simulink model "isi_correlator"  (Simulink 7.0)
settings: Solver=ode45  StartTime=0.0  StopTime=2500  RelTol=1e-3
undefined symbols (not defined in the file): bit_rev, pfb_coeff_gen_calc
libraries: xbsIndex_r4 x4490, casper_library x399, xbsReplacements_r4 x27, isi_correlator_lib x8, xps_library x7, dspsigops x2, simulink x1
(part 1 of 49)
settings Model: Creator=wjm  LastModifiedBy=wjm

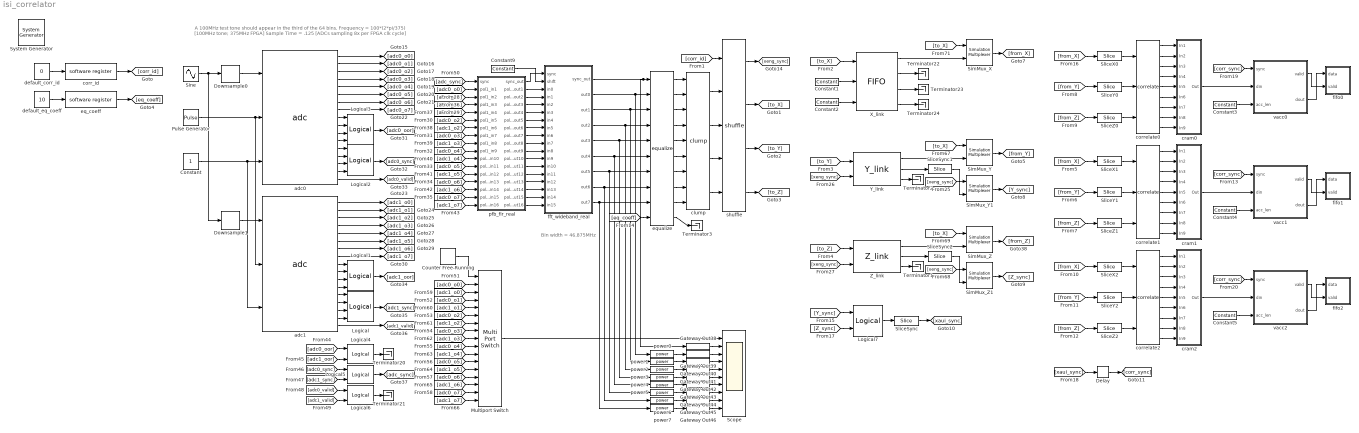
== system isi_correlator ==
  note: A 100MHz test tone should appear in the third of the 64 bins.
      | Frequency = 100*(2*pi/375) [100MHz tone; 375MHz FPGA]
      | Sample Time = .125 [ADCs sampling 8x per FPGA clk cycle]
  note: Bin width = 46.875MHz
  " System Generator" : xbsIndex_r4/ System Generator  Tag=genX  SystemSampleTime=-1  xilinxfamily=virtex5  part=xc5vsx95t  speed=-1  package=ff1136  synthesis_tool=XST  clock_wrapper=Clock Enables  directory=./isi_correlator/sysgen  testbench=off  simulink_period=1  sysclk_period=2.6667  dcm_input_clock_period=100  incr_netlist=off  trim_vbits=Everywhere in SubSystem  dbl_ovrd=According to Block Masks  core_generation=According to Block Masks  run_coregen=off  deprecated_control=off  eval_field=0  block_type=sysgen
      settings: block_version=10.1.1
  Constant : Constant  OutDataType=sfix(16)  OutScaling=2^0  SampleTime=1
  Constant1 : xbsIndex_r4/Constant  arith_type=Boolean  const=1  n_bits=1  bin_pt=0  explicit_period=on  period=1  equ=P=C  opselect=C  inp2=PCIN>>17  opr=+  inp1=P  carry=CIN  dbl_ovrd=off  block_type=constant
      settings: block_version=10.1.1
  Constant2 : xbsIndex_r4/Constant  arith_type=Boolean  const=1  n_bits=1  bin_pt=0  explicit_period=on  period=1  equ=P=C  opselect=C  inp2=PCIN>>17  opr=+  inp1=P  carry=CIN  dbl_ovrd=off  block_type=constant
      settings: block_version=10.1.1
  Constant3 : xbsIndex_r4/Constant  arith_type=Unsigned  const=127  n_bits=8  bin_pt=0  explicit_period=on  period=1  equ=P=C  opselect=C  inp2=PCIN>>17  opr=+  inp1=P  carry=CIN  dbl_ovrd=off  block_type=constant
      settings: block_version=10.1.1
  Constant4 : xbsIndex_r4/Constant  arith_type=Unsigned  const=127  n_bits=8  bin_pt=0  explicit_period=on  period=1  equ=P=C  opselect=C  inp2=PCIN>>17  opr=+  inp1=P  carry=CIN  dbl_ovrd=off  block_type=constant
      settings: block_version=10.1.1
  Constant5 : xbsIndex_r4/Constant  arith_type=Unsigned  const=127  n_bits=8  bin_pt=0  explicit_period=on  period=1  equ=P=C  opselect=C  inp2=PCIN>>17  opr=+  inp1=P  carry=CIN  dbl_ovrd=off  block_type=constant
      settings: block_version=10.1.1
  Constant9 : xbsIndex_r4/Constant  arith_type=Unsigned  const=127  n_bits=7  bin_pt=0  explicit_period=on  period=1  equ=P=C  opselect=C  inp2=PCIN>>17  opr=+  inp1=P  carry=CIN  dbl_ovrd=off  block_type=constant
      settings: block_version=10.1.1
  Counter Free-Running : simulink/Sources/Counter Free-Running  SystemSampleTime=-1  NumBits=4  tsamp=1/16
  Delay : xbsIndex_r4/Delay  en=off  latency=1  dbl_ovrd=off  reg_retiming=off  block_type=delay
  Downsample0 : dspsigops/Downsample  N=2  phase=1  ic=0  smode=Allow multirate  fmode=Maintain input frame size
  Downsample1 : dspsigops/Downsample  N=2  phase=0  ic=0  smode=Allow multirate  fmode=Maintain input frame size
  Gateway Out{38..46} (9 alike) : xbsIndex_r4/Gateway Out  hdl_port=on  timing_constraint=None  locs_specified=off  LOCs={}  block_type=gatewayout
      settings: infoedit="Gateway out block.  Converts Xilinx fixed point inputs into ouputs of type Simulink integer, double, or fixed point.<P><P>Hardware notes:  In hardware these blocks become top level output ports or are discarded, depending on how they are configured."  block_version=10.1.3
  Logical{,1..3} (4 alike) : xbsIndex_r4/Logical  logical_function=OR  inputs=4  en=off  latency=0  precision=Full  arith_type=Unsigned  n_bits=1  bin_pt=0  align_bp=on  dbl_ovrd=off  block_type=logical
      settings: block_version=10.1.1
  Logical4 : xbsIndex_r4/Logical  logical_function=OR  inputs=2  en=off  latency=0  precision=Full  arith_type=Unsigned  n_bits=1  bin_pt=0  align_bp=on  dbl_ovrd=off  block_type=logical
      settings: block_version=10.1.1
  Logical5 : xbsIndex_r4/Logical  logical_function=OR  inputs=2  en=off  latency=0  precision=Full  arith_type=Unsigned  n_bits=1  bin_pt=0  align_bp=on  dbl_ovrd=off  block_type=logical
      settings: block_version=10.1.1
  Logical6 : xbsIndex_r4/Logical  logical_function=AND  inputs=2  en=off  latency=0  precision=Full  arith_type=Unsigned  n_bits=1  bin_pt=0  align_bp=on  dbl_ovrd=off  block_type=logical
      settings: block_version=10.1.1
  Logical7 : xbsIndex_r4/Logical  logical_function=OR  inputs=2  en=off  latency=0  precision=Full  arith_type=Unsigned  n_bits=16  bin_pt=0  align_bp=on  dbl_ovrd=off  block_type=logical
  Multiport Switch : MultiPortSwitch  Inputs=16  zeroidx=on  OutDataType=sfix(16)  OutScaling=2^0
  Pulse Generator : DiscretePulseGenerator  Period=2^12
  Scope : Scope  NumInputPorts=10
      settings: YMin=-1~-1~-1~0~-8.13152e-020~0~0~0~0~0  YMax=1~1~1~0.00275~0.0014~0.00175~0.00175~0.00225~0.00175~0.00225
  SimMux_X : xbsIndex_r4/Simulation Multiplexer  sim_sel=1  hw_sel=2  block_type=simmux
      settings: infoedit="Distinguish input subsystems as \"simulation only\" and \"generation only\".  The input specified For Simulation will be used during Simulink simulation.  The input specified For Generation will be used during code generation.  This block will typically be used on the output of a HW Co-Simulation, Black Box, or ModelSim subsystem.<P><P>Hardware Notes: This block costs nothing."  block_version=10.1.3
  SimMux_Y : xbsIndex_r4/Simulation Multiplexer  sim_sel=1  hw_sel=2  block_type=simmux
      settings: infoedit="Distinguish input subsystems as \"simulation only\" and \"generation only\".  The input specified For Simulation will be used during Simulink simulation.  The input specified For Generation will be used during code generation.  This block will typically be used on the output of a HW Co-Simulation, Black Box, or ModelSim subsystem.<P><P>Hardware Notes: This block costs nothing."  block_version=10.1.3
  SimMux_Y1 : xbsIndex_r4/Simulation Multiplexer  sim_sel=1  hw_sel=2  block_type=simmux
      settings: infoedit="Distinguish input subsystems as \"simulation only\" and \"generation only\".  The input specified For Simulation will be used during Simulink simulation.  The input specified For Generation will be used during code generation.  This block will typically be used on the output of a HW Co-Simulation, Black Box, or ModelSim subsystem.<P><P>Hardware Notes: This block costs nothing."  block_version=10.1.3
  SimMux_Z : xbsIndex_r4/Simulation Multiplexer  sim_sel=1  hw_sel=2  block_type=simmux
      settings: infoedit="Distinguish input subsystems as \"simulation only\" and \"generation only\".  The input specified For Simulation will be used during Simulink simulation.  The input specified For Generation will be used during code generation.  This block will typically be used on the output of a HW Co-Simulation, Black Box, or ModelSim subsystem.<P><P>Hardware Notes: This block costs nothing."  block_version=10.1.3
  SimMux_Z1 : xbsIndex_r4/Simulation Multiplexer  sim_sel=1  hw_sel=2  block_type=simmux
      settings: infoedit="Distinguish input subsystems as \"simulation only\" and \"generation only\".  The input specified For Simulation will be used during Simulink simulation.  The input specified For Generation will be used during code generation.  This block will typically be used on the output of a HW Co-Simulation, Black Box, or ModelSim subsystem.<P><P>Hardware Notes: This block costs nothing."  block_version=10.1.3
  Sine : Sin  Amplitude=.9  Frequency=100*(2*pi/375)  SampleTime=1/16
  SliceSync : xbsIndex_r4/Slice  nbits=8  boolean_output=on  mode=Lower Bit Location + Width  bit1=0  base1=MSB of Input  bit0=0  base0=LSB of Input  dbl_ovrd=off  block_type=slice
      settings: infoedit="Extracts a given range of bits from each input sample and presents it at the output.  The output type is ordinarily unsigned with binary point at zero, but can be Boolean when the slice is one bit wide.<P><P>Hardware notes: In hardware this block costs nothing."  block_version=10.1.1
  SliceSync1 : xbsIndex_r4/Slice  nbits=8  boolean_output=on  mode=Lower Bit Location + Width  bit1=0  base1=MSB of Input  bit0=0  base0=LSB of Input  dbl_ovrd=off  block_type=slice
      settings: infoedit="Extracts a given range of bits from each input sample and presents it at the output.  The output type is ordinarily unsigned with binary point at zero, but can be Boolean when the slice is one bit wide.<P><P>Hardware notes: In hardware this block costs nothing."  block_version=10.1.1
  SliceSync2 : xbsIndex_r4/Slice  nbits=8  boolean_output=on  mode=Lower Bit Location + Width  bit1=0  base1=MSB of Input  bit0=0  base0=LSB of Input  dbl_ovrd=off  block_type=slice
      settings: infoedit="Extracts a given range of bits from each input sample and presents it at the output.  The output type is ordinarily unsigned with binary point at zero, but can be Boolean when the slice is one bit wide.<P><P>Hardware notes: In hardware this block costs nothing."  block_version=10.1.1
  SliceX0 : xbsIndex_r4/Slice  nbits=8  boolean_output=off  mode=Upper Bit Location + Width  bit1=0  base1=MSB of Input  bit0=0  base0=LSB of Input  dbl_ovrd=off  block_type=slice
      settings: infoedit="Extracts a given range of bits from each input sample and presents it at the output.  The output type is ordinarily unsigned with binary point at zero, but can be Boolean when the slice is one bit wide.<P><P>Hardware notes: In hardware this block costs nothing."  block_version=10.1.1
  SliceX1 : xbsIndex_r4/Slice  nbits=8  boolean_output=off  mode=Upper Bit Location + Width  bit1=-8  base1=MSB of Input  bit0=8  base0=LSB of Input  dbl_ovrd=off  block_type=slice
      settings: infoedit="Extracts a given range of bits from each input sample and presents it at the output.  The output type is ordinarily unsigned with binary point at zero, but can be Boolean when the slice is one bit wide.<P><P>Hardware notes: In hardware this block costs nothing."  block_version=10.1.1
  SliceX2 : xbsIndex_r4/Slice  nbits=8  boolean_output=off  mode=Upper Bit Location + Width  bit1=-16  base1=MSB of Input  bit0=16  base0=LSB of Input  dbl_ovrd=off  block_type=slice
      settings: infoedit="Extracts a given range of bits from each input sample and presents it at the output.  The output type is ordinarily unsigned with binary point at zero, but can be Boolean when the slice is one bit wide.<P><P>Hardware notes: In hardware this block costs nothing."  block_version=10.1.1
  SliceY0 : xbsIndex_r4/Slice  nbits=8  boolean_output=off  mode=Upper Bit Location + Width  bit1=0  base1=MSB of Input  bit0=0  base0=LSB of Input  dbl_ovrd=off  block_type=slice
      settings: infoedit="Extracts a given range of bits from each input sample and presents it at the output.  The output type is ordinarily unsigned with binary point at zero, but can be Boolean when the slice is one bit wide.<P><P>Hardware notes: In hardware this block costs nothing."  block_version=10.1.1
  SliceY1 : xbsIndex_r4/Slice  nbits=8  boolean_output=off  mode=Upper Bit Location + Width  bit1=-8  base1=MSB of Input  bit0=8  base0=LSB of Input  dbl_ovrd=off  block_type=slice
      settings: infoedit="Extracts a given range of bits from each input sample and presents it at the output.  The output type is ordinarily unsigned with binary point at zero, but can be Boolean when the slice is one bit wide.<P><P>Hardware notes: In hardware this block costs nothing."  block_version=10.1.1
  SliceY2 : xbsIndex_r4/Slice  nbits=8  boolean_output=off  mode=Upper Bit Location + Width  bit1=-16  base1=MSB of Input  bit0=16  base0=LSB of Input  dbl_ovrd=off  block_type=slice
      settings: infoedit="Extracts a given range of bits from each input sample and presents it at the output.  The output type is ordinarily unsigned with binary point at zero, but can be Boolean when the slice is one bit wide.<P><P>Hardware notes: In hardware this block costs nothing."  block_version=10.1.1
  SliceZ0 : xbsIndex_r4/Slice  nbits=8  boolean_output=off  mode=Upper Bit Location + Width  bit1=0  base1=MSB of Input  bit0=0  base0=LSB of Input  dbl_ovrd=off  block_type=slice
      settings: infoedit="Extracts a given range of bits from each input sample and presents it at the output.  The output type is ordinarily unsigned with binary point at zero, but can be Boolean when the slice is one bit wide.<P><P>Hardware notes: In hardware this block costs nothing."  block_version=10.1.1
  SliceZ1 : xbsIndex_r4/Slice  nbits=8  boolean_output=off  mode=Upper Bit Location + Width  bit1=-8  base1=MSB of Input  bit0=8  base0=LSB of Input  dbl_ovrd=off  block_type=slice
      settings: infoedit="Extracts a given range of bits from each input sample and presents it at the output.  The output type is ordinarily unsigned with binary point at zero, but can be Boolean when the slice is one bit wide.<P><P>Hardware notes: In hardware this block costs nothing."  block_version=10.1.1
  SliceZ2 : xbsIndex_r4/Slice  nbits=8  boolean_output=off  mode=Upper Bit Location + Width  bit1=-16  base1=MSB of Input  bit0=16  base0=LSB of Input  dbl_ovrd=off  block_type=slice
      settings: infoedit="Extracts a given range of bits from each input sample and presents it at the output.  The output type is ordinarily unsigned with binary point at zero, but can be Boolean when the slice is one bit wide.<P><P>Hardware notes: In hardware this block costs nothing."  block_version=10.1.1
  Terminator{1..3,20..24} (8 alike) : Terminator
  X_link : xbsIndex_r4/FIFO  depth=16  percent_nbits=1  rst=off  en=off  use_dcount=off  use_almost_empty=off  almost_empty_thresh=2  use_almost_full=off  almost_full_thresh=14  dbl_ovrd=off  mem_type=Block RAM  block_type=fifo
      settings: block_version=10.1.1
  Y_link : isi_correlator_lib/Y_link  SystemSampleTime=-1
      link override XAUI0/convert_tx_data: n_bits=32
      link override XAUI0/convert_tx_outofband: n_bits=4
      link override XAUI0/isi_corr_Y_link_XAUI0_rx_data: n_bits=32
      link override XAUI0/isi_corr_Y_link_XAUI0_rx_outofband: n_bits=4
      link override XAUI0/isi_correlator_Y_link_XAUI0_rx_data: n_bits=32
      link override XAUI0/isi_correlator_Y_link_XAUI0_rx_outofband: n_bits=4
      link override XAUI1/convert_tx_data: n_bits=32
      link override XAUI1/convert_tx_outofband: n_bits=4
      link override XAUI1/isi_corr_Y_link_XAUI1_rx_data: n_bits=32
      link override XAUI1/isi_corr_Y_link_XAUI1_rx_outofband: n_bits=4
      link override XAUI1/isi_correlator_Y_link_XAUI1_rx_data: n_bits=32
      link override XAUI1/isi_correlator_Y_link_XAUI1_rx_outofband: n_bits=4
  Z_link : isi_correlator_lib/Z_link  SystemSampleTime=-1
      link override XAUI2/convert_tx_data: n_bits=32
      link override XAUI2/convert_tx_outofband: n_bits=4
      link override XAUI2/isi_corr_Z_link_XAUI2_rx_data: n_bits=32
      link override XAUI2/isi_corr_Z_link_XAUI2_rx_outofband: n_bits=4
      link override XAUI2/isi_correlator_Z_link_XAUI2_rx_data: n_bits=32
      link override XAUI2/isi_correlator_Z_link_XAUI2_rx_outofband: n_bits=4
      link override XAUI3/convert_tx_data: n_bits=32
      link override XAUI3/convert_tx_outofband: n_bits=4
      link override XAUI3/isi_corr_Z_link_XAUI3_rx_data: n_bits=32
      link override XAUI3/isi_corr_Z_link_XAUI3_rx_outofband: n_bits=4
      link override XAUI3/isi_correlator_Z_link_XAUI3_rx_data: n_bits=32
      link override XAUI3/isi_correlator_Z_link_XAUI3_rx_outofband: n_bits=4
  adc0 : xps_library/adc  Tag=xps:adc  SystemSampleTime=-1  adc_brd=adc0  adc_clk_rate=1500  adc_interleave=on  sample_period=1
  adc1 : xps_library/adc  Tag=xps:adc  SystemSampleTime=-1  adc_brd=adc1  adc_clk_rate=1500  adc_interleave=on  sample_period=1
  clump : isi_correlator_lib/clump  SystemSampleTime=-1
  corr_id : xps_library/software register  Tag=xps:sw_reg  SystemSampleTime=-1  io_dir=From Processor  arith_type=Unsigned  bitwidth=32  bin_pt=0  sample_period=1  gw_name=isi_correlator_corr_id_user_data_out
      link override corr_3ghz_10_1_corr_id_user_data_out: arith_type=Unsigned  n_bits=32  bin_pt=0  period=1
      link override corr_3ghz_10_1_exp_corr_id_user_data_out: arith_type=Unsigned  n_bits=32  bin_pt=0  period=1
      link override isi_corr_corr_id_user_data_out: arith_type=Unsigned  n_bits=32  bin_pt=0  period=1
      link override isi_correlator_corr_id_user_data_out: arith_type=Unsigned  n_bits=32  bin_pt=0  period=1
  correlate0 : isi_correlator_lib/correlate  SystemSampleTime=-1
  correlate1 : isi_correlator_lib/correlate  SystemSampleTime=-1
  correlate2 : isi_correlator_lib/correlate  SystemSampleTime=-1
  cram0 : Subsystem  AncestorBlock=gavrt_library/cram  mask(cram){num_slice=9}
      mask prompts: num_slice "Number of slices"
      mask help: Concatenates inputs together after forcing binary point to zero.
      mask init:
      | cram_init(gcb, ...
      |     'num_slice', num_slice);
  cram1 : Subsystem  AncestorBlock=gavrt_library/cram  mask(cram){num_slice=9}
      mask prompts: num_slice "Number of slices"
      mask help: Concatenates inputs together after forcing binary point to zero.
      mask init:
      | cram_init(gcb, ...
      |     'num_slice', num_slice);
  cram2 : Subsystem  AncestorBlock=gavrt_library/cram  mask(cram){num_slice=9}
      mask prompts: num_slice "Number of slices"
      mask help: Concatenates inputs together after forcing binary point to zero.
      mask init:
      | cram_init(gcb, ...
      |     'num_slice', num_slice);
  default_corr_id : Constant  Value=0  OutDataType=sfix(16)  OutScaling=2^0  SampleTime=1
  default_eq_coeff : Constant  Value=10  OutDataType=sfix(16)  OutScaling=2^0  SampleTime=1
  eq_coeff : xps_library/software register  Tag=xps:sw_reg  SystemSampleTime=-1  io_dir=From Processor  arith_type=Unsigned  bitwidth=32  bin_pt=0  sample_period=1  gw_name=isi_correlator_eq_coeff_user_data_out
      link override corr_3ghz_10_1_corr_id_user_data_out: arith_type=Unsigned  n_bits=32  bin_pt=0  period=1
      link override corr_3ghz_10_1_exp_corr_id_user_data_out: arith_type=Unsigned  n_bits=32  bin_pt=0  period=1
      link override isi_corr_eq_coeff_user_data_out: arith_type=Unsigned  n_bits=32  bin_pt=0  period=1
      link override isi_correlator_eq_coeff_user_data_out: arith_type=Unsigned  n_bits=32  bin_pt=0  period=1
  equalize : isi_correlator_lib/equalize  SystemSampleTime=-1
  fft_wideband_real : Subsystem  AncestorBlock=casper_library/FFTs/fft_wideband_real  mask(fft_wideband_real){FFTSize=7, BitWidth=18, n_inputs=4, quantization=Truncate, overflow=Wrap, add_latency=2, mult_latency=2, bram_latency=3}
      mask prompts: FFTSize "Size of FFT: (2^?)"; BitWidth "Bit Width"; n_inputs "Number of Simultaneous Inputs: (2^?)"; quantization "Quantization Behavior"; overflow "Overflow Behavior"; add_latency "Add Latency"; mult_latency "Mult Latency"; bram_latency "BRAM Latency"
      mask init:
      | fft_wideband_real_init(gcb,...
      |     'FFTSize', FFTSize,...
      |     'BitWidth', BitWidth,...
      |     'n_inputs', n_inputs,...
      |     'quantization', quantization,...
      |     'overflow', overflow,...
      |     'add_latency', add_latency,...
      |     'mult_latency', mult_latency,...
      |     'bram_latency', bram_latency);
      settings: AttributesFormatString=FFTSize=7, n_inputs=4
      mask StyleString: edit,edit,edit,popup(Truncate|Round  (unbiased: +/- Inf)|Round  (unbiased: Even Values)),popup(Wrap|Saturate|Error),edit,edit,edit
  fifo0 : Subsystem  AncestorBlock=isi_correlator_lib/fifo
  fifo1 : Subsystem  AncestorBlock=isi_correlator_lib/fifo
  fifo2 : Subsystem  AncestorBlock=isi_correlator_lib/fifo
  pfb_fir_real : Subsystem  AncestorBlock=casper_library/PFBs/pfb_fir_real  mask(pfb_fir_real){PFBSize=8, TotalTaps=4, WindowType=hamming, n_inputs=4, MakeBiplex=0, BitWidthIn=8, BitWidthOut=18, CoeffBitWidth=18, CoeffDistMem=0, add_latency=1, mult_latency=2, bram_latency=2, quantization=Truncate, fwidth=1}
      mask prompts: PFBSize "Size of PFB: (2^? pnts)"; TotalTaps "Total Number of Taps:"; WindowType "Windowing Function:"; n_inputs "Number of Simultaneous Inputs: (2^?)"; MakeBiplex "Make Biplex"; BitWidthIn "Input Bitwidth:"; BitWidthOut "Output Bitwidth:"; CoeffBitWidth "Coefficient Bitwidth:"; CoeffDistMem "Use Distributed Memory for Coeffs"; add_latency "Add Latency"; mult_latency "Mult Latency"; bram_latency "BRAM Latency"; quantization "Quantization Behavior"; fwidth "Bin Width Scaling (normal=1)"
      mask init:
      | pfb_fir_real_init(gcb,...
      |     'PFBSize', PFBSize,...
      |     'TotalTaps', TotalTaps,...
      |     'WindowType', WindowType,...
      |     'n_inputs', n_inputs,...
      |     'MakeBiplex', MakeBiplex,...
      |     'BitWidthIn', BitWidthIn,...
      |     'BitWidthOut', BitWidthOut,...
      |     'CoeffBitWidth', CoeffBitWidth,...
      |     'CoeffDistMem', CoeffDistMem,...
      |     'add_latency', add_latency,...
      |     'mult_latency', mult_latency,...
      |     'bram_latency', bram_latency,...
      |     'quantization', quantization,...
      |     'fwidth', fwidth);
      settings: AttributesFormatString=taps=4, add_latency=1
      mask Help: eval('xlWeb([getenv(''MLIB_ROOT''), ''\doc\htmlblocks\sp_pfb_fir_real\sp_pfb_fir_real.html''])')
      mask StyleString: edit,edit,popup(bartlett|barthannwin|blackman|blackmanharris|bohamwin|chebwin|flattopwin|gausswin|hamming|hann|kaiser|nuttallwin|parzenwin|rectwin|tukeywin|triang|userwindow),edit,edit,edit,edit,edit,edit,edit,edit,edit,popup(Truncate|Round  (unbiased: +/- Inf)|Round  (unbiased: Even Values)),edit
  power{0..7} (8 alike) : casper_library/Misc/power  SystemSampleTime=-1  BitWidth=18
  shuffle : isi_correlator_lib/shuffle  SystemSampleTime=-1
  vacc0 : Subsystem  AncestorBlock=gavrt_library/vacc  mask(vacc){veclen=3, ninputs=9, max_accum=7, arith_type=1, in_bit_width=8, in_bin_pt=7, out_bit_width=28, out_bin_pt=7, register_din=1, add_latency=2, bram_latency=2, mux_latency=1}
      mask prompts: veclen "Vector Length (2^?)"; ninputs "Number of Simultaneous Vectors"; max_accum "Maximum # Accumulations (2^?)"; arith_type "Arithmetic Type (0=Unsigned, 1=Signed)"; in_bit_width "Input Bit Width"; in_bin_pt "Input Binary Point"; out_bit_width "Output Bit Width"; out_bin_pt "Output Binary Point"; register_din "Register Input Data  (1 = Enable Register Retiming on input delay)"; add_latency "Add Latency"; bram_latency "BRAM Latency"; mux_latency "Mux Latency"
      mask help:
      | Sync is expected the clock before the first data to be accumulated.
      | Use the Cram block to feed din for multiple simultaneous inputs.
      | acc_len is the number of vectors to sum.
      | valid is high while a valid accumulation is being clocked out on dout.
      | If only one sync pulse is provided, valid accumulations will repeatedly appear on dout as they become available.
      | Undesired behavior may occur if acc_len is changed without a new sync pulse.
      | Note: The output is an unsigned concatenation of the output streams. Thus, even with only one stream, the output must be recast before using.
      | Register Input Data controls the "Enable Register Retiming" parameter of the delays on 'din'.
      mask init:
      | vacc_init(gcb, ...
      |     'veclen', veclen, ...
      |     'ninputs', ninputs, ...
      |     'max_accum', max_accum, ...
      |     'arith_type', arith_type, ...
      |     'in_bit_width', in_bit_width, ...
      |     'in_bin_pt', in_bin_pt, ...
      |     'out_bit_width', out_bit_width, ...
      |     'out_bin_pt', out_bin_pt, ...
      |     'register_din', register_din, ...
      |     'add_latency', add_latency, ...
      |     'bram_latency', bram_latency, ...
      |     'mux_latency', mux_latency);
      settings: AttributesFormatString=|
      | vector length=8, inputs=9
      | max accumulations=2^7
  vacc1 : Subsystem  AncestorBlock=gavrt_library/vacc  mask(vacc){veclen=3, ninputs=9, max_accum=7, arith_type=1, in_bit_width=8, in_bin_pt=7, out_bit_width=28, out_bin_pt=7, register_din=1, add_latency=2, bram_latency=2, mux_latency=1}
      mask prompts: veclen "Vector Length (2^?)"; ninputs "Number of Simultaneous Vectors"; max_accum "Maximum # Accumulations (2^?)"; arith_type "Arithmetic Type (0=Unsigned, 1=Signed)"; in_bit_width "Input Bit Width"; in_bin_pt "Input Binary Point"; out_bit_width "Output Bit Width"; out_bin_pt "Output Binary Point"; register_din "Register Input Data  (1 = Enable Register Retiming on input delay)"; add_latency "Add Latency"; bram_latency "BRAM Latency"; mux_latency "Mux Latency"
      mask help:
      | Sync is expected the clock before the first data to be accumulated.
      | Use the Cram block to feed din for multiple simultaneous inputs.
      | acc_len is the number of vectors to sum.
      | valid is high while a valid accumulation is being clocked out on dout.
      | If only one sync pulse is provided, valid accumulations will repeatedly appear on dout as they become available.
      | Undesired behavior may occur if acc_len is changed without a new sync pulse.
      | Note: The output is an unsigned concatenation of the output streams. Thus, even with only one stream, the output must be recast before using.
      | Register Input Data controls the "Enable Register Retiming" parameter of the delays on 'din'.
      mask init: same as isi_correlator/vacc0
      settings: AttributesFormatString=|
      | vector length=8, inputs=9
      | max accumulations=2^7
  vacc2 : Subsystem  AncestorBlock=gavrt_library/vacc  mask(vacc){veclen=3, ninputs=9, max_accum=7, arith_type=1, in_bit_width=8, in_bin_pt=7, out_bit_width=28, out_bin_pt=7, register_din=1, add_latency=2, bram_latency=2, mux_latency=1}
      mask prompts: veclen "Vector Length (2^?)"; ninputs "Number of Simultaneous Vectors"; max_accum "Maximum # Accumulations (2^?)"; arith_type "Arithmetic Type (0=Unsigned, 1=Signed)"; in_bit_width "Input Bit Width"; in_bin_pt "Input Binary Point"; out_bit_width "Output Bit Width"; out_bin_pt "Output Binary Point"; register_din "Register Input Data  (1 = Enable Register Retiming on input delay)"; add_latency "Add Latency"; bram_latency "BRAM Latency"; mux_latency "Mux Latency"
      mask help:
      | Sync is expected the clock before the first data to be accumulated.
      | Use the Cram block to feed din for multiple simultaneous inputs.
      | acc_len is the number of vectors to sum.
      | valid is high while a valid accumulation is being clocked out on dout.
      | If only one sync pulse is provided, valid accumulations will repeatedly appear on dout as they become available.
      | Undesired behavior may occur if acc_len is changed without a new sync pulse.
      | Note: The output is an unsigned concatenation of the output streams. Thus, even with only one stream, the output must be recast before using.
      | Register Input Data controls the "Enable Register Retiming" parameter of the delays on 'din'.
      mask init: same as isi_correlator/vacc0
      settings: AttributesFormatString=|
      | vector length=8, inputs=9
      | max accumulations=2^7
  wires:
  adc1/9 -> Logical1/1
  adc1/10 -> Logical1/2
  adc1/11 -> Logical1/3
  adc1/12 -> Logical1/4
  adc1/13 -> Logical/1
  adc1/14 -> Logical/2
  adc1/15 -> Logical/3
  adc1/16 -> Logical/4
  fft_wideband_real/2(out0) -> equalize/2, power0/1
  fft_wideband_real/3(out1) -> equalize/3, power1/1
  fft_wideband_real/4(out2) -> equalize/4, power2/1
  fft_wideband_real/5(out3) -> equalize/5, power3/1
  fft_wideband_real/6(out4) -> equalize/6, power4/1
  fft_wideband_real/7(out5) -> equalize/7, power5/1
  fft_wideband_real/8(out6) -> equalize/8, power6/1
  fft_wideband_real/9(out7) -> equalize/9, power7/1
  corr_id/1 -> [corr_id]
  default_corr_id/1 -> corr_id/1
  Z_link/1 -> SimMux_Z/2
  [from_X] -> SliceX0/1
  [from_Y] -> SliceY0/1
  [from_Z] -> SliceZ0/1
  fft_wideband_real/1(sync_out) -> equalize/1, Gateway Out38/1
  [from_X] -> SliceX1/1
  [from_Y] -> SliceY1/1
  [from_Z] -> SliceZ1/1
  [from_X] -> SliceX2/1
  [from_Y] -> SliceY2/1
  [from_Z] -> SliceZ2/1
  Gateway Out38/1 -> Scope/2
  Gateway Out39/1 -> Scope/3
  power0/1 -> Gateway Out39/1
  power1/1 -> Gateway Out40/1
  power2/1 -> Gateway Out41/1
  power3/1 -> Gateway Out42/1
  power4/1 -> Gateway Out43/1
  power5/1 -> Gateway Out44/1
  power6/1 -> Gateway Out45/1
  power7/1 -> Gateway Out46/1
  Gateway Out40/1 -> Scope/4
  Gateway Out41/1 -> Scope/5
  Gateway Out42/1 -> Scope/6
  Gateway Out43/1 -> Scope/7
  Gateway Out44/1 -> Scope/8
  Gateway Out45/1 -> Scope/9
  Gateway Out46/1 -> Scope/10
  adc0/9 -> Logical3/1
  adc0/10 -> Logical3/2
  adc0/11 -> Logical3/3
  adc0/12 -> Logical3/4
  adc0/1 -> [adc0_o0]
  adc0/2 -> [adc0_o1]
  adc0/3 -> [adc0_o2]
  adc0/4 -> [adc0_o3]
  adc0/5 -> [adc0_o4]
  adc0/6 -> [adc0_o5]
  adc0/7 -> [adc0_o6]
  adc0/8 -> [adc0_o7]
  adc1/1 -> [adc1_o0]
  adc1/2 -> [adc1_o1]
  adc1/3 -> [adc1_o2]
  adc1/4 -> [adc1_o3]
  adc1/5 -> [adc1_o4]
  adc1/6 -> [adc1_o5]
  adc1/7 -> [adc1_o6]
  adc1/8 -> [adc1_o7]
  Pulse Generator/1 -> adc0/2, adc1/2
  Constant/1 -> adc0/3, adc1/3
  Sine/1 -> Downsample0/1, Downsample1/1
  adc0/13 -> Logical2/1
  adc0/14 -> Logical2/2
  adc0/15 -> Logical2/3
  adc0/16 -> Logical2/4
  Logical2/1 -> [adc0_sync]
  adc0/17 -> [adc0_valid]
  Logical3/1 -> [adc0_oor]
  Logical1/1 -> [adc1_oor]
  adc1/17 -> [adc1_valid]
  Logical/1 -> [adc1_sync]
  [adc0_oor] -> Logical4/1
  [adc1_oor] -> Logical4/2
  [adc0_sync] -> Logical5/1
  [adc1_sync] -> Logical5/2
  [adc0_valid] -> Logical6/1
  [adc1_valid] -> Logical6/2
  Logical4/1 -> Terminator20/1
  Logical6/1 -> Terminator21/1
  Logical5/1 -> [adc_sync]
  Downsample0/1 -> adc0/1
  Downsample1/1 -> adc1/1
  [adc_sync] -> pfb_fir_real/1(sync)
  [adc0_o0] -> pfb_fir_real/2(pol1_in1)
  [adc1_o0] -> pfb_fir_real/3(pol1_in2)
  [adc0_o1] -> pfb_fir_real/4(pol1_in3)
  [adc1_o1] -> pfb_fir_real/5(pol1_in4)
  [adc0_o2] -> pfb_fir_real/6(pol1_in5)
  [adc1_o2] -> pfb_fir_real/7(pol1_in6)
  [adc0_o3] -> pfb_fir_real/8(pol1_in7)
  [adc1_o3] -> pfb_fir_real/9(pol1_in8)
  [adc0_o4] -> pfb_fir_real/10(pol1_in9)
  [adc1_o4] -> pfb_fir_real/11(pol1_in10)
  [adc0_o5] -> pfb_fir_real/12(pol1_in11)
  [adc1_o5] -> pfb_fir_real/13(pol1_in12)
  [adc0_o6] -> pfb_fir_real/14(pol1_in13)
  [adc1_o6] -> pfb_fir_real/15(pol1_in14)
  [adc0_o7] -> pfb_fir_real/16(pol1_in15)
  [adc1_o7] -> pfb_fir_real/17(pol1_in16)
  pfb_fir_real/2(pol1_out1) -> fft_wideband_real/3(in0)
  pfb_fir_real/3(pol1_out2) -> fft_wideband_real/4(in1)
  pfb_fir_real/4(pol1_out3) -> fft_wideband_real/5(in2)
  pfb_fir_real/5(pol1_out4) -> fft_wideband_real/6(in3)
  pfb_fir_real/6(pol1_out5) -> fft_wideband_real/7(in4)
  pfb_fir_real/7(pol1_out6) -> fft_wideband_real/8(in5)
  pfb_fir_real/8(pol1_out7) -> fft_wideband_real/9(in6)
  pfb_fir_real/9(pol1_out8) -> fft_wideband_real/10(in7)
  pfb_fir_real/10(pol1_out9) -> fft_wideband_real/11(in8)
  pfb_fir_real/11(pol1_out10) -> fft_wideband_real/12(in9)
  pfb_fir_real/12(pol1_out11) -> fft_wideband_real/13(in10)
  pfb_fir_real/13(pol1_out12) -> fft_wideband_real/14(in11)
  pfb_fir_real/14(pol1_out13) -> fft_wideband_real/15(in12)
  pfb_fir_real/15(pol1_out14) -> fft_wideband_real/16(in13)
  pfb_fir_real/16(pol1_out15) -> fft_wideband_real/17(in14)
  pfb_fir_real/17(pol1_out16) -> fft_wideband_real/18(in15)
  Counter Free-Running/1 -> Multiport Switch/1
  [adc0_o0] -> Multiport Switch/2
  [adc1_o0] -> Multiport Switch/3
  [adc0_o1] -> Multiport Switch/4
  [adc1_o1] -> Multiport Switch/5
  [adc0_o2] -> Multiport Switch/6
  [adc1_o2] -> Multiport Switch/7
  [adc0_o3] -> Multiport Switch/8
  [adc1_o3] -> Multiport Switch/9
  [adc0_o4] -> Multiport Switch/10
  [adc1_o4] -> Multiport Switch/11
  [adc0_o5] -> Multiport Switch/12
  [adc1_o5] -> Multiport Switch/13
  [adc0_o6] -> Multiport Switch/14
  [adc1_o6] -> Multiport Switch/15
  [adc0_o7] -> Multiport Switch/16
  SimMux_Y/1 -> [from_Y]
  [to_X] -> SimMux_Y/1
  SimMux_Z/1 -> [from_Z]
  [to_X] -> SimMux_Z/1
  [to_X] -> X_link/1
  Constant2/1 -> X_link/3
  X_link/1 -> SimMux_X/2
  X_link/2 -> Terminator22/1
  X_link/3 -> Terminator23/1
  X_link/4 -> Terminator24/1
  SimMux_X/1 -> [from_X]
  [to_X] -> SimMux_X/1
  equalize/1 -> clump/1
  equalize/2 -> clump/2
  equalize/3 -> clump/3
  equalize/4 -> clump/4
  equalize/5 -> clump/5
  equalize/6 -> clump/6
  equalize/7 -> clump/7
  equalize/8 -> clump/8
  equalize/9 -> clump/9
  clump/1 -> shuffle/2
  clump/2 -> shuffle/3
  clump/3 -> shuffle/4
  clump/4 -> shuffle/5
  shuffle/1 -> [xeng_sync]
  shuffle/2 -> [to_X]
  shuffle/3 -> [to_Y]
  shuffle/4 -> [to_Z]
  Constant1/1 -> X_link/2
  Y_link/1 -> SimMux_Y/2
  equalize/10 -> Terminator3/1
  eq_coeff/1 -> [eq_coeff]
  default_eq_coeff/1 -> eq_coeff/1
  [eq_coeff] -> equalize/10
  [corr_id] -> shuffle/1
  [adc1_o7] -> Multiport Switch/17
  Multiport Switch/1 -> Scope/1
  SliceX0/1 -> correlate0/1
  SliceY0/1 -> correlate0/2
  SliceZ0/1 -> correlate0/3
  SliceX1/1 -> correlate1/1
  SliceY1/1 -> correlate1/2
  SliceZ1/1 -> correlate1/3
  SliceX2/1 -> correlate2/1
  SliceY2/1 -> correlate2/2
  SliceZ2/1 -> correlate2/3
  correlate0/1 -> cram0/1(In1)
  correlate0/2 -> cram0/2(In2)
  correlate0/3 -> cram0/3(In3)
  correlate0/4 -> cram0/4(In4)
  correlate0/5 -> cram0/5(In5)
  correlate0/6 -> cram0/6(In6)
  correlate0/7 -> cram0/7(In7)
  correlate0/8 -> cram0/8(In8)
  correlate0/9 -> cram0/9(In9)
  correlate1/1 -> cram1/1(In1)
  correlate1/2 -> cram1/2(In2)
  correlate1/3 -> cram1/3(In3)
  correlate1/4 -> cram1/4(In4)
  correlate1/5 -> cram1/5(In5)
  correlate1/6 -> cram1/6(In6)
  correlate1/7 -> cram1/7(In7)
  correlate1/8 -> cram1/8(In8)
  correlate1/9 -> cram1/9(In9)
  correlate2/1 -> cram2/1(In1)
  correlate2/2 -> cram2/2(In2)
  correlate2/3 -> cram2/3(In3)
  correlate2/4 -> cram2/4(In4)
  correlate2/5 -> cram2/5(In5)
  correlate2/6 -> cram2/6(In6)
  correlate2/7 -> cram2/7(In7)
  correlate2/8 -> cram2/8(In8)
  correlate2/9 -> cram2/9(In9)
  Constant3/1 -> vacc0/3(acc_len)
  [to_Z] -> Z_link/1
  [xeng_sync] -> Z_link/2
  Z_link/2 -> SliceSync2/1
  Y_link/2 -> SliceSync1/1
  Y_link/3 -> Terminator2/1
  Z_link/3 -> Terminator1/1
  [to_Y] -> Y_link/1
  [xeng_sync] -> Y_link/2
  [Y_sync] -> Logical7/1
  [Z_sync] -> Logical7/2
  [xaui_sync] -> Delay/1
  Delay/1 -> [corr_sync]
  [corr_sync] -> vacc0/1(sync)
  SliceSync/1 -> [xaui_sync]
  Logical7/1 -> SliceSync/1
  [xeng_sync] -> SimMux_Y1/1
  SimMux_Y1/1 -> [Y_sync]
  SimMux_Z1/1 -> [Z_sync]
  [xeng_sync] -> SimMux_Z1/1
  SliceSync1/1 -> SimMux_Y1/2
  SliceSync2/1 -> SimMux_Z1/2
  Constant4/1 -> vacc1/3(acc_len)
  [corr_sync] -> vacc1/1(sync)
  Constant5/1 -> vacc2/3(acc_len)
  [corr_sync] -> vacc2/1(sync)
  cram2/1(Out) -> vacc2/2(din)
  cram1/1(Out) -> vacc1/2(din)
  cram0/1(Out) -> vacc0/2(din)
  vacc2/1(valid) -> fifo2/2(valid)
  vacc1/1(valid) -> fifo1/2(valid)
  vacc0/1(valid) -> fifo0/2(valid)
  vacc0/2(dout) -> fifo0/1(data)
  vacc1/2(dout) -> fifo1/1(data)
  vacc2/2(dout) -> fifo2/1(data)
  pfb_fir_real/1(sync_out) -> fft_wideband_real/1(sync)
  Constant9/1 -> fft_wideband_real/2(shift)

== system isi_correlator/cram0 ==
  In1 : Inport  OutDataType=sfix(16)  OutScaling=2^0
  In2 : Inport  Port=2  OutDataType=sfix(16)  OutScaling=2^0
  In3 : Inport  Port=3  OutDataType=sfix(16)  OutScaling=2^0
  In4 : Inport  Port=4
  In5 : Inport  Port=5
  In6 : Inport  Port=6
  In7 : Inport  Port=7
  In8 : Inport  Port=8
  In9 : Inport  Port=9
  Concat : xbsIndex_r4/Concat  num_inputs=9  dbl_ovrd=off  block_type=concat
      settings: block_version=10.1.2
  Reinterp{1..9} (9 alike) : xbsIndex_r4/Reinterpret  force_arith_type=on  arith_type=Unsigned  force_bin_pt=on  bin_pt=0  block_type=reinterpret
      Reinterp1 settings: infoedit="Changes signal type without altering the binary representation.   You can changed the signal between signed and unsigned, and relocate the binary point.<P><P>Hardware notes: In hardware this block costs nothing.<P><P>Example:  Suppose the input is 6 bits wide, signed, with 2 fractional bits, and the output is forced to unsigned with 0 fractional bits.  Then an input of -2.0 (1110.00 in binary 2's complement) becomes an output of 56 (111000 in binary)."  block_version=10.1.2
      Reinterp2 settings: infoedit="Changes signal type without altering the binary representation.   You can changed the signal between signed and unsigned, and relocate the binary point.<P><P>Hardware notes: In hardware this block costs nothing.<P><P>Example:  Suppose the input is 6 bits wide, signed, with 2 fractional bits, and the output is forced to unsigned with 0 fractional bits.  Then an input of -2.0 (1110.00 in binary 2's complement) becomes an output of 56 (111000 in binary)."  block_version=10.1.2
      Reinterp3 settings: infoedit="Changes signal type without altering the binary representation.   You can changed the signal between signed and unsigned, and relocate the binary point.<P><P>Hardware notes: In hardware this block costs nothing.<P><P>Example:  Suppose the input is 6 bits wide, signed, with 2 fractional bits, and the output is forced to unsigned with 0 fractional bits.  Then an input of -2.0 (1110.00 in binary 2's complement) becomes an output of 56 (111000 in binary)."  block_version=10.1.2
      Reinterp4 settings: infoedit="Changes signal type without altering the binary representation.   You can changed the signal between signed and unsigned, and relocate the binary point.<P><P>Hardware notes: In hardware this block costs nothing.<P><P>Example:  Suppose the input is 6 bits wide, signed, with 2 fractional bits, and the output is forced to unsigned with 0 fractional bits.  Then an input of -2.0 (1110.00 in binary 2's complement) becomes an output of 56 (111000 in binary)."  block_version=10.1.2
      Reinterp5 settings: infoedit="Changes signal type without altering the binary representation.   You can changed the signal between signed and unsigned, and relocate the binary point.<P><P>Hardware notes: In hardware this block costs nothing.<P><P>Example:  Suppose the input is 6 bits wide, signed, with 2 fractional bits, and the output is forced to unsigned with 0 fractional bits.  Then an input of -2.0 (1110.00 in binary 2's complement) becomes an output of 56 (111000 in binary)."  block_version=10.1.3
      Reinterp6 settings: infoedit="Changes signal type without altering the binary representation.   You can changed the signal between signed and unsigned, and relocate the binary point.<P><P>Hardware notes: In hardware this block costs nothing.<P><P>Example:  Suppose the input is 6 bits wide, signed, with 2 fractional bits, and the output is forced to unsigned with 0 fractional bits.  Then an input of -2.0 (1110.00 in binary 2's complement) becomes an output of 56 (111000 in binary)."  block_version=10.1.3
      Reinterp7 settings: infoedit="Changes signal type without altering the binary representation.   You can changed the signal between signed and unsigned, and relocate the binary point.<P><P>Hardware notes: In hardware this block costs nothing.<P><P>Example:  Suppose the input is 6 bits wide, signed, with 2 fractional bits, and the output is forced to unsigned with 0 fractional bits.  Then an input of -2.0 (1110.00 in binary 2's complement) becomes an output of 56 (111000 in binary)."  block_version=10.1.3
      Reinterp8 settings: infoedit="Changes signal type without altering the binary representation.   You can changed the signal between signed and unsigned, and relocate the binary point.<P><P>Hardware notes: In hardware this block costs nothing.<P><P>Example:  Suppose the input is 6 bits wide, signed, with 2 fractional bits, and the output is forced to unsigned with 0 fractional bits.  Then an input of -2.0 (1110.00 in binary 2's complement) becomes an output of 56 (111000 in binary)."  block_version=10.1.3
      Reinterp9 settings: infoedit="Changes signal type without altering the binary representation.   You can changed the signal between signed and unsigned, and relocate the binary point.<P><P>Hardware notes: In hardware this block costs nothing.<P><P>Example:  Suppose the input is 6 bits wide, signed, with 2 fractional bits, and the output is forced to unsigned with 0 fractional bits.  Then an input of -2.0 (1110.00 in binary 2's complement) becomes an output of 56 (111000 in binary)."  block_version=10.1.3
  Out : Outport
  wires:
  Concat/1 -> Out/1
  In1/1 -> Reinterp1/1
  Reinterp1/1 -> Concat/1
  In2/1 -> Reinterp2/1
  Reinterp2/1 -> Concat/2
  In3/1 -> Reinterp3/1
  Reinterp3/1 -> Concat/3
  In4/1 -> Reinterp4/1
  Reinterp4/1 -> Concat/4
  In5/1 -> Reinterp5/1
  Reinterp5/1 -> Concat/5
  In6/1 -> Reinterp6/1
  Reinterp6/1 -> Concat/6
  In7/1 -> Reinterp7/1
  Reinterp7/1 -> Concat/7
  In8/1 -> Reinterp8/1
  Reinterp8/1 -> Concat/8
  In9/1 -> Reinterp9/1
  Reinterp9/1 -> Concat/9

== system isi_correlator/cram1 ==
  In1 : Inport  OutDataType=sfix(16)  OutScaling=2^0
  In2 : Inport  Port=2  OutDataType=sfix(16)  OutScaling=2^0
  In3 : Inport  Port=3  OutDataType=sfix(16)  OutScaling=2^0
  In4 : Inport  Port=4
  In5 : Inport  Port=5
  In6 : Inport  Port=6
  In7 : Inport  Port=7
  In8 : Inport  Port=8
  In9 : Inport  Port=9
  Concat : xbsIndex_r4/Concat  num_inputs=9  dbl_ovrd=off  block_type=concat
      settings: block_version=10.1.2
  Reinterp{1..9} (9 alike) : xbsIndex_r4/Reinterpret  force_arith_type=on  arith_type=Unsigned  force_bin_pt=on  bin_pt=0  block_type=reinterpret
      Reinterp1 settings: infoedit="Changes signal type without altering the binary representation.   You can changed the signal between signed and unsigned, and relocate the binary point.<P><P>Hardware notes: In hardware this block costs nothing.<P><P>Example:  Suppose the input is 6 bits wide, signed, with 2 fractional bits, and the output is forced to unsigned with 0 fractional bits.  Then an input of -2.0 (1110.00 in binary 2's complement) becomes an output of 56 (111000 in binary)."  block_version=10.1.2
      Reinterp2 settings: infoedit="Changes signal type without altering the binary representation.   You can changed the signal between signed and unsigned, and relocate the binary point.<P><P>Hardware notes: In hardware this block costs nothing.<P><P>Example:  Suppose the input is 6 bits wide, signed, with 2 fractional bits, and the output is forced to unsigned with 0 fractional bits.  Then an input of -2.0 (1110.00 in binary 2's complement) becomes an output of 56 (111000 in binary)."  block_version=10.1.2
      Reinterp3 settings: infoedit="Changes signal type without altering the binary representation.   You can changed the signal between signed and unsigned, and relocate the binary point.<P><P>Hardware notes: In hardware this block costs nothing.<P><P>Example:  Suppose the input is 6 bits wide, signed, with 2 fractional bits, and the output is forced to unsigned with 0 fractional bits.  Then an input of -2.0 (1110.00 in binary 2's complement) becomes an output of 56 (111000 in binary)."  block_version=10.1.2
      Reinterp4 settings: infoedit="Changes signal type without altering the binary representation.   You can changed the signal between signed and unsigned, and relocate the binary point.<P><P>Hardware notes: In hardware this block costs nothing.<P><P>Example:  Suppose the input is 6 bits wide, signed, with 2 fractional bits, and the output is forced to unsigned with 0 fractional bits.  Then an input of -2.0 (1110.00 in binary 2's complement) becomes an output of 56 (111000 in binary)."  block_version=10.1.2
      Reinterp5 settings: infoedit="Changes signal type without altering the binary representation.   You can changed the signal between signed and unsigned, and relocate the binary point.<P><P>Hardware notes: In hardware this block costs nothing.<P><P>Example:  Suppose the input is 6 bits wide, signed, with 2 fractional bits, and the output is forced to unsigned with 0 fractional bits.  Then an input of -2.0 (1110.00 in binary 2's complement) becomes an output of 56 (111000 in binary)."  block_version=10.1.3
      Reinterp6 settings: infoedit="Changes signal type without altering the binary representation.   You can changed the signal between signed and unsigned, and relocate the binary point.<P><P>Hardware notes: In hardware this block costs nothing.<P><P>Example:  Suppose the input is 6 bits wide, signed, with 2 fractional bits, and the output is forced to unsigned with 0 fractional bits.  Then an input of -2.0 (1110.00 in binary 2's complement) becomes an output of 56 (111000 in binary)."  block_version=10.1.3
      Reinterp7 settings: infoedit="Changes signal type without altering the binary representation.   You can changed the signal between signed and unsigned, and relocate the binary point.<P><P>Hardware notes: In hardware this block costs nothing.<P><P>Example:  Suppose the input is 6 bits wide, signed, with 2 fractional bits, and the output is forced to unsigned with 0 fractional bits.  Then an input of -2.0 (1110.00 in binary 2's complement) becomes an output of 56 (111000 in binary)."  block_version=10.1.3
      Reinterp8 settings: infoedit="Changes signal type without altering the binary representation.   You can changed the signal between signed and unsigned, and relocate the binary point.<P><P>Hardware notes: In hardware this block costs nothing.<P><P>Example:  Suppose the input is 6 bits wide, signed, with 2 fractional bits, and the output is forced to unsigned with 0 fractional bits.  Then an input of -2.0 (1110.00 in binary 2's complement) becomes an output of 56 (111000 in binary)."  block_version=10.1.3
      Reinterp9 settings: infoedit="Changes signal type without altering the binary representation.   You can changed the signal between signed and unsigned, and relocate the binary point.<P><P>Hardware notes: In hardware this block costs nothing.<P><P>Example:  Suppose the input is 6 bits wide, signed, with 2 fractional bits, and the output is forced to unsigned with 0 fractional bits.  Then an input of -2.0 (1110.00 in binary 2's complement) becomes an output of 56 (111000 in binary)."  block_version=10.1.3
  Out : Outport
  wires:
  Concat/1 -> Out/1
  In1/1 -> Reinterp1/1
  Reinterp1/1 -> Concat/1
  In2/1 -> Reinterp2/1
  Reinterp2/1 -> Concat/2
  In3/1 -> Reinterp3/1
  Reinterp3/1 -> Concat/3
  In4/1 -> Reinterp4/1
  Reinterp4/1 -> Concat/4
  In5/1 -> Reinterp5/1
  Reinterp5/1 -> Concat/5
  In6/1 -> Reinterp6/1
  Reinterp6/1 -> Concat/6
  In7/1 -> Reinterp7/1
  Reinterp7/1 -> Concat/7
  In8/1 -> Reinterp8/1
  Reinterp8/1 -> Concat/8
  In9/1 -> Reinterp9/1
  Reinterp9/1 -> Concat/9
